simulink model "CCMHfaMotor"  (R2022b)
settings: Solver=FixedStepDiscrete  StartTime=0.0  StopTime=10.0  FixedStep=0.01  RelTol=1e-3
model workspace (MAT data in the model file):
  HfaWakeUpFlag : Simulink.Signal (value not decoded)
  NvMBlockHfaMtrCalData : Simulink.Signal (value not decoded)
  NvMBlockHfaMtrCalDataInit : Simulink.Parameter (value not decoded)
symbols that are objects in the model workspace (value not decoded): NvMBlockHfaMtrCalDataInit
undefined symbols (not defined in the file or in its folder): ComChlType, DioChannelType, HFAChlType, LinFrameType, NetworkChlType, NvMBlockId, P_DioLevelType, P_LinIfSchHandleType, P_Std_ReturnType, PowerChlType
(part 2 of 4)

== system CCMHfaMotor/CCMHfaMotor_HfaMotMode_sys ==
  function : TriggerPort  TriggerType=function-call  VariantControl=(inherit)
  PA_NvMBlockHfaMtrCalDataInit : Constant  Value=NvMBlockHfaMtrCalDataInit
  Terminator_1 : Terminator
  wires:
  PA_NvMBlockHfaMtrCalDataInit/1 -> Terminator_1/1

== system CCMHfaMotor/CCMHfaMotor_ReHvacMotMode_sys ==
  function : TriggerPort  TriggerType=function-call  VariantControl=(inherit)
  PA_NvMBlockHfaMtrCalDataInit : Constant  Value=NvMBlockHfaMtrCalDataInit
  Terminator_1 : Terminator
  wires:
  PA_NvMBlockHfaMtrCalDataInit/1 -> Terminator_1/1

== system CCMHfaMotor/CCMHfaMotor_WtrMotMode_sys ==
  function : TriggerPort  TriggerType=function-call  VariantControl=(inherit)
  PA_NvMBlockHfaMtrCalDataInit : Constant  Value=NvMBlockHfaMtrCalDataInit
  Terminator_1 : Terminator
  wires:
  PA_NvMBlockHfaMtrCalDataInit/1 -> Terminator_1/1

== system CCMHfaMotor/HfaCaliService ==
  HfaCaliService_SetAllCalibrate_sys : Subsystem
  HfaCaliService_SetAllParaInit_sys : Subsystem
  HfaCaliService_SetMotorCalibrate_sys : Subsystem
  HfaCaliService_SetMotorParaInit_sys : Subsystem

== system CCMHfaMotor/HfaDtcService ==
  HfaDtcService_GetElecErr_sys : Subsystem
  HfaDtcService_GetRecErr_sys : Subsystem
  HfaDtcService_GetStallErr_sys : Subsystem
  HfaDtcService_GetTempErr_sys : Subsystem
  HfaDtcService_GetVoltErr_sys : Subsystem

== system CCMHfaMotor/HfaIOCtrlService ==
  HfaIOCtrlService_FreezeMotorCurrentPosition_sys : Subsystem
  HfaIOCtrlService_MotorReturnToECU_sys : Subsystem
  HfaIOCtrlService_MotorShortTermAdj_sys : Subsystem

== system CCMHfaMotor/HfaMotor_50msRunnable_sys ==
  function : TriggerPort  TriggerType=function-call  VariantControl=(inherit)  SampleTimeType=periodic  SampleTime=-1
  PA_NvMBlockHfaMtrCalDataInit : Constant  Value=NvMBlockHfaMtrCalDataInit
  Terminator_1 : Terminator
  wires:
  PA_NvMBlockHfaMtrCalDataInit/1 -> Terminator_1/1

== system CCMHfaMotor/HfaMotor_Init ==
  SbcPwrOn_SbcPwrOn_read : Inport
  SbcWakeupSta_SbcWakeupSta_read : Inport  Port=2
  Event Listener : EventListener  EventName=reset
  PA_NvMBlockHfaMtrCalDataInit : Constant  Value=NvMBlockHfaMtrCalDataInit
  Terminator_1 : Terminator
  Terminator_2 : Terminator
  Terminator_3 : Terminator
  wires:
  SbcPwrOn_SbcPwrOn_read/1 -> Terminator_1/1
  SbcWakeupSta_SbcWakeupSta_read/1 -> Terminator_2/1
  PA_NvMBlockHfaMtrCalDataInit/1 -> Terminator_3/1
